simulink model "hyd_hyb_2_opt_cont_test_new_1_col_ab"  (Simulink 7.7)
settings: Solver=ode14x  StartTime=0  StopTime=750  FixedStep=0.01  MaxStep=.4  RelTol=1e-8
InitFcn:
  | const_3
values from neighbouring files (symbols this model uses):
  Af = 6.3    [brk_enrg.m]    (also in brk_enrg_1.m)
  BSFC_906_205 = [3.5684 4.3466 5.1668 6.0769 6.988 7.8992 8.8104 9.7336 10.8548 11.9809 13.1112 14.2571 ... (663 elements, 39x17)]    [BSFC_map_906_205.mat]
  Cd = 0.55    [brk_enrg.m]    (also in brk_enrg_1.m)
  den = 1.202    [brk_enrg.m]    (also in brk_enrg_1.m)
  fr = 0.01    [brk_enrg.m]    (also in brk_enrg_1.m)
undefined symbols (not defined in the file or in its folder): clutchRise, down_spd_2, down_spd_3, down_spd_4, down_spd_c, fuel_density, fuz_wgt, gearshift, I_wheel, Idle_spd_eng_906, M1_hydr, p_v_15, pmax_125, qv_125, Rw, sec_eng_sfc_map, T_max_906, T_min, TC_spd_ratio_max, torque_convertor, Twait, up_spd_1, up_spd_2, up_spd_3, up_spd_c, v_p_10, v_p_15, v_p_5
libraries: neural x2963, fl_lib x43, nesl_utility x30, sdl_lib x14, simulink x4, simulink_need_slupdate x1
(part 72 of 72)

== system hyd_hyb_2_opt_cont_test_new_1_col_ab/controller1/optimized controller - pm/SOC upper 0.5/SOC upper 0.6/SOC upper 0.7/SOC upper 0.8/net_pm_col_ab_89_b/Layer 1/IW{1,1} ==
  "pd{1,1}" : Inport  PortDimensions=2
      mask Display: e = 0.06;c1 = [120 230 180]/255;c2 = ([120 230 180]+0)/(256+128);c3 = ([120 230 180]+128)/(256+128);patch([0 1 1 0],[0 0 1 1],c1);patch([0 1 1 1-e 1-e 0+e],[0 0 1 1-e 0+e 0+e],c2);patch([0 0 1 1-e 0+e 0+e],[0 1 1 1-e 1-e 0+e],c3);plot([0 1 1 0 0],[0 0 1 1 0]);
  "IW{1,1}(1,:)'" : Constant  Value=[-7.5247531718272755;-6.6194856288513249]
      mask Display: e = 0.06;c1 = [120 150 230]/255;c2 = ([120 150 230]+0)/383;c3 = ([120 150 230]+128)/383;patch([0 1 1 0],[0 0 1 1],c1);patch([0 1 1 1-e 1-e 0+e],[0 0 1 1-e 0+e 0+e],c2);patch([0 0 1 1-e 0+e 0+e],[0 1 1 1-e 1-e 0+e],c3);plot([0 1 1 0 0],[0 0 1 1 0]);disp('Weights');
  "IW{1,1}(10,:)'" : Constant  Value=[-0.6823961158945111;-10.925155075088925]
      mask Display: e = 0.06;c1 = [120 150 230]/255;c2 = ([120 150 230]+0)/383;c3 = ([120 150 230]+128)/383;patch([0 1 1 0],[0 0 1 1],c1);patch([0 1 1 1-e 1-e 0+e],[0 0 1 1-e 0+e 0+e],c2);patch([0 0 1 1-e 0+e 0+e],[0 1 1 1-e 1-e 0+e],c3);plot([0 1 1 0 0],[0 0 1 1 0]);disp('Weights');
  "IW{1,1}(11,:)'" : Constant  Value=[10.664799059669335;0.99738837220633847]
      mask Display: e = 0.06;c1 = [120 150 230]/255;c2 = ([120 150 230]+0)/383;c3 = ([120 150 230]+128)/383;patch([0 1 1 0],[0 0 1 1],c1);patch([0 1 1 1-e 1-e 0+e],[0 0 1 1-e 0+e 0+e],c2);patch([0 0 1 1-e 0+e 0+e],[0 1 1 1-e 1-e 0+e],c3);plot([0 1 1 0 0],[0 0 1 1 0]);disp('Weights');
  "IW{1,1}(12,:)'" : Constant  Value=[1.8521265658009105;12.123277674207074]
      mask Display: e = 0.06;c1 = [120 150 230]/255;c2 = ([120 150 230]+0)/383;c3 = ([120 150 230]+128)/383;patch([0 1 1 0],[0 0 1 1],c1);patch([0 1 1 1-e 1-e 0+e],[0 0 1 1-e 0+e 0+e],c2);patch([0 0 1 1-e 0+e 0+e],[0 1 1 1-e 1-e 0+e],c3);plot([0 1 1 0 0],[0 0 1 1 0]);disp('Weights');
  "IW{1,1}(13,:)'" : Constant  Value=[9.8761699255633211;8.8585443365510503]
      mask Display: e = 0.06;c1 = [120 150 230]/255;c2 = ([120 150 230]+0)/383;c3 = ([120 150 230]+128)/383;patch([0 1 1 0],[0 0 1 1],c1);patch([0 1 1 1-e 1-e 0+e],[0 0 1 1-e 0+e 0+e],c2);patch([0 0 1 1-e 0+e 0+e],[0 1 1 1-e 1-e 0+e],c3);plot([0 1 1 0 0],[0 0 1 1 0]);disp('Weights');
  "IW{1,1}(14,:)'" : Constant  Value=[-5.3819119390644117;-7.2068768579593456]
      mask Display: e = 0.06;c1 = [120 150 230]/255;c2 = ([120 150 230]+0)/383;c3 = ([120 150 230]+128)/383;patch([0 1 1 0],[0 0 1 1],c1);patch([0 1 1 1-e 1-e 0+e],[0 0 1 1-e 0+e 0+e],c2);patch([0 0 1 1-e 0+e 0+e],[0 1 1 1-e 1-e 0+e],c3);plot([0 1 1 0 0],[0 0 1 1 0]);disp('Weights');
  "IW{1,1}(2,:)'" : Constant  Value=[3.4569355142303508;-9.9292384009218217]
      mask Display: e = 0.06;c1 = [120 150 230]/255;c2 = ([120 150 230]+0)/383;c3 = ([120 150 230]+128)/383;patch([0 1 1 0],[0 0 1 1],c1);patch([0 1 1 1-e 1-e 0+e],[0 0 1 1-e 0+e 0+e],c2);patch([0 0 1 1-e 0+e 0+e],[0 1 1 1-e 1-e 0+e],c3);plot([0 1 1 0 0],[0 0 1 1 0]);disp('Weights');
  "IW{1,1}(3,:)'" : Constant  Value=[-12.547076601117634;-1.3578183381190847]
      mask Display: e = 0.06;c1 = [120 150 230]/255;c2 = ([120 150 230]+0)/383;c3 = ([120 150 230]+128)/383;patch([0 1 1 0],[0 0 1 1],c1);patch([0 1 1 1-e 1-e 0+e],[0 0 1 1-e 0+e 0+e],c2);patch([0 0 1 1-e 0+e 0+e],[0 1 1 1-e 1-e 0+e],c3);plot([0 1 1 0 0],[0 0 1 1 0]);disp('Weights');
  "IW{1,1}(4,:)'" : Constant  Value=[-8.5225171072598229;-5.570962150913628]
      mask Display: e = 0.06;c1 = [120 150 230]/255;c2 = ([120 150 230]+0)/383;c3 = ([120 150 230]+128)/383;patch([0 1 1 0],[0 0 1 1],c1);patch([0 1 1 1-e 1-e 0+e],[0 0 1 1-e 0+e 0+e],c2);patch([0 0 1 1-e 0+e 0+e],[0 1 1 1-e 1-e 0+e],c3);plot([0 1 1 0 0],[0 0 1 1 0]);disp('Weights');
  "IW{1,1}(5,:)'" : Constant  Value=[-10.165810742362757;-2.8900949275636489]
      mask Display: e = 0.06;c1 = [120 150 230]/255;c2 = ([120 150 230]+0)/383;c3 = ([120 150 230]+128)/383;patch([0 1 1 0],[0 0 1 1],c1);patch([0 1 1 1-e 1-e 0+e],[0 0 1 1-e 0+e 0+e],c2);patch([0 0 1 1-e 0+e 0+e],[0 1 1 1-e 1-e 0+e],c3);plot([0 1 1 0 0],[0 0 1 1 0]);disp('Weights');
  "IW{1,1}(6,:)'" : Constant  Value=[-2.1735501686188834;-10.275369821335833]
      mask Display: e = 0.06;c1 = [120 150 230]/255;c2 = ([120 150 230]+0)/383;c3 = ([120 150 230]+128)/383;patch([0 1 1 0],[0 0 1 1],c1);patch([0 1 1 1-e 1-e 0+e],[0 0 1 1-e 0+e 0+e],c2);patch([0 0 1 1-e 0+e 0+e],[0 1 1 1-e 1-e 0+e],c3);plot([0 1 1 0 0],[0 0 1 1 0]);disp('Weights');
  "IW{1,1}(7,:)'" : Constant  Value=[-9.29204133005614;-5.9530925476551584]
      mask Display: e = 0.06;c1 = [120 150 230]/255;c2 = ([120 150 230]+0)/383;c3 = ([120 150 230]+128)/383;patch([0 1 1 0],[0 0 1 1],c1);patch([0 1 1 1-e 1-e 0+e],[0 0 1 1-e 0+e 0+e],c2);patch([0 0 1 1-e 0+e 0+e],[0 1 1 1-e 1-e 0+e],c3);plot([0 1 1 0 0],[0 0 1 1 0]);disp('Weights');
  "IW{1,1}(8,:)'" : Constant  Value=[8.790230610730708;6.6353593624284661]
      mask Display: e = 0.06;c1 = [120 150 230]/255;c2 = ([120 150 230]+0)/383;c3 = ([120 150 230]+128)/383;patch([0 1 1 0],[0 0 1 1],c1);patch([0 1 1 1-e 1-e 0+e],[0 0 1 1-e 0+e 0+e],c2);patch([0 0 1 1-e 0+e 0+e],[0 1 1 1-e 1-e 0+e],c3);plot([0 1 1 0 0],[0 0 1 1 0]);disp('Weights');
  "IW{1,1}(9,:)'" : Constant  Value=[6.7949067036882491;-8.3979254167496382]
      mask Display: e = 0.06;c1 = [120 150 230]/255;c2 = ([120 150 230]+0)/383;c3 = ([120 150 230]+128)/383;patch([0 1 1 0],[0 0 1 1],c1);patch([0 1 1 1-e 1-e 0+e],[0 0 1 1-e 0+e 0+e],c2);patch([0 0 1 1-e 0+e 0+e],[0 1 1 1-e 1-e 0+e],c3);plot([0 1 1 0 0],[0 0 1 1 0]);disp('Weights');
  Mux : Mux  Inputs=14
      mask Display: e = 0.06;c1 = [120 150 230]/255;c2 = ([120 150 230]+0)/383;c3 = ([120 150 230]+128)/383;patch([0 1 1 0],[0 0 1 1],c1);patch([0 1 1 1-e 1-e 0+e],[0 0 1 1-e 0+e 0+e],c2);patch([0 0 1 1-e 0+e 0+e],[0 1 1 1-e 1-e 0+e],c3);plot([0 1 1 0 0],[0 0 1 1 0]);disp('Mux');
  dotprod{1..14} (14 alike) : neural/Weight Functions/dotprod  SystemSampleTime=-1
  "iz{1,1}" : Outport
      mask Display: e = 0.06;c1 = [120 230 180]/255;c2 = ([120 230 180]+0)/(256+128);c3 = ([120 230 180]+128)/(256+128);patch([0 1 1 0],[0 0 1 1],c1);patch([0 1 1 1-e 1-e 0+e],[0 0 1 1-e 0+e 0+e],c2);patch([0 0 1 1-e 0+e 0+e],[0 1 1 1-e 1-e 0+e],c3);plot([0 1 1 0 0],[0 0 1 1 0]);
  wires:
  "IW{1,1}(1,:)'"/1 -> dotprod1/1
  "pd{1,1}"/1 -> dotprod1/2, dotprod2/2, dotprod3/2, dotprod4/2, dotprod5/2, dotprod6/2, dotprod7/2, dotprod8/2, dotprod9/2, dotprod10/2, dotprod11/2, dotprod12/2, dotprod13/2, dotprod14/2
  dotprod1/1 -> Mux/1
  "IW{1,1}(2,:)'"/1 -> dotprod2/1
  dotprod2/1 -> Mux/2
  "IW{1,1}(3,:)'"/1 -> dotprod3/1
  dotprod3/1 -> Mux/3
  "IW{1,1}(4,:)'"/1 -> dotprod4/1
  dotprod4/1 -> Mux/4
  "IW{1,1}(5,:)'"/1 -> dotprod5/1
  dotprod5/1 -> Mux/5
  "IW{1,1}(6,:)'"/1 -> dotprod6/1
  dotprod6/1 -> Mux/6
  "IW{1,1}(7,:)'"/1 -> dotprod7/1
  dotprod7/1 -> Mux/7
  "IW{1,1}(8,:)'"/1 -> dotprod8/1
  dotprod8/1 -> Mux/8
  "IW{1,1}(9,:)'"/1 -> dotprod9/1
  dotprod9/1 -> Mux/9
  "IW{1,1}(10,:)'"/1 -> dotprod10/1
  dotprod10/1 -> Mux/10
  "IW{1,1}(11,:)'"/1 -> dotprod11/1
  dotprod11/1 -> Mux/11
  "IW{1,1}(12,:)'"/1 -> dotprod12/1
  dotprod12/1 -> Mux/12
  "IW{1,1}(13,:)'"/1 -> dotprod13/1
  dotprod13/1 -> Mux/13
  "IW{1,1}(14,:)'"/1 -> dotprod14/1
  dotprod14/1 -> Mux/14
  Mux/1 -> "iz{1,1}"/1

== system hyd_hyb_2_opt_cont_test_new_1_col_ab/controller1/optimized controller - pm/SOC upper 0.5/SOC upper 0.6/SOC upper 0.7/SOC upper 0.8/net_pm_col_ab_89_b/Layer 2/Delays 1 ==
  "p{2}" : Inport  PortDimensions=14
      mask Display: e = 0.06;c1 = [120 230 180]/255;c2 = ([120 230 180]+0)/(256+128);c3 = ([120 230 180]+128)/(256+128);patch([0 1 1 0],[0 0 1 1],c1);patch([0 1 1 1-e 1-e 0+e],[0 0 1 1-e 0+e 0+e],c2);patch([0 0 1 1-e 0+e 0+e],[0 1 1 1-e 1-e 0+e],c3);plot([0 1 1 0 0],[0 0 1 1 0]);
  mux : Mux  Inputs=1
      mask Display: e = 0.06;c1 = [120 150 230]/255;c2 = ([120 150 230]+0)/383;c3 = ([120 150 230]+128)/383;patch([0 1 1 0],[0 0 1 1],c1);patch([0 1 1 1-e 1-e 0+e],[0 0 1 1-e 0+e 0+e],c2);patch([0 0 1 1-e 0+e 0+e],[0 1 1 1-e 1-e 0+e],c3);plot([0 1 1 0 0],[0 0 1 1 0]);disp('Mux')
  "pd{2,1}" : Outport
      mask Display: e = 0.06;c1 = [120 230 180]/255;c2 = ([120 230 180]+0)/(256+128);c3 = ([120 230 180]+128)/(256+128);patch([0 1 1 0],[0 0 1 1],c1);patch([0 1 1 1-e 1-e 0+e],[0 0 1 1-e 0+e 0+e],c2);patch([0 0 1 1-e 0+e 0+e],[0 1 1 1-e 1-e 0+e],c3);plot([0 1 1 0 0],[0 0 1 1 0]);
  wires:
  "p{2}"/1 -> mux/1
  mux/1 -> "pd{2,1}"/1

== system hyd_hyb_2_opt_cont_test_new_1_col_ab/controller1/optimized controller - pm/SOC upper 0.5/SOC upper 0.6/SOC upper 0.7/SOC upper 0.8/net_pm_col_ab_89_b/Layer 2/LW{2,1} ==
  "ad{2,1}" : Inport  PortDimensions=14
      mask Display: e = 0.06;c1 = [120 230 180]/255;c2 = ([120 230 180]+0)/(256+128);c3 = ([120 230 180]+128)/(256+128);patch([0 1 1 0],[0 0 1 1],c1);patch([0 1 1 1-e 1-e 0+e],[0 0 1 1-e 0+e 0+e],c2);patch([0 0 1 1-e 0+e 0+e],[0 1 1 1-e 1-e 0+e],c3);plot([0 1 1 0 0],[0 0 1 1 0]);
  "IW{2,1}(1,:)'" : Constant  Value=[-2.4172362230861912;0.11897161735180822;1.0748128173288254;1.8399688966148295;-1.4152398719051704;1.2831233160485713;0.66619502504356864;-3.7040294434188823;-1.6806920562993684;1.4872763390073449;0.22853571508898307;2.8622448895932617;1.6734974110640288;2.3644811190421393]
      mask Display: e = 0.06;c1 = [120 150 230]/255;c2 = ([120 150 230]+0)/383;c3 = ([120 150 230]+128)/383;patch([0 1 1 0],[0 0 1 1],c1);patch([0 1 1 1-e 1-e 0+e],[0 0 1 1-e 0+e 0+e],c2);patch([0 0 1 1-e 0+e 0+e],[0 1 1 1-e 1-e 0+e],c3);plot([0 1 1 0 0],[0 0 1 1 0]);disp('weights');
  "IW{2,1}(10,:)'" : Constant  Value=[2.1725003018942353;-2.9655421023585693;-1.9087526907654899;0.066652972634019494;-1.1505504360832728;-2.348205137749996;-2.6986676089208288;-3.134462355153997;-2.4105504942557419;-3.5078854741284391;-0.73741384502139795;-0.65533890123243521;-1.9470593645380398;-2.4696809181554595]
      mask Display: e = 0.06;c1 = [120 150 230]/255;c2 = ([120 150 230]+0)/383;c3 = ([120 150 230]+128)/383;patch([0 1 1 0],[0 0 1 1],c1);patch([0 1 1 1-e 1-e 0+e],[0 0 1 1-e 0+e 0+e],c2);patch([0 0 1 1-e 0+e 0+e],[0 1 1 1-e 1-e 0+e],c3);plot([0 1 1 0 0],[0 0 1 1 0]);disp('weights');
  "IW{2,1}(11,:)'" : Constant  Value=[-2.5933643387457002;2.5545627202222185;2.211227347756926;2.4888386205217095;-2.2126597028366959;-1.6982454335324346;1.1168846576098335;-1.6225169470611134;0.065669659624881027;-1.6044919910126278;-2.1834160484889371;-1.4830708033907178;-2.6046033852372132;0.60418726384841592]
      mask Display: e = 0.06;c1 = [120 150 230]/255;c2 = ([120 150 230]+0)/383;c3 = ([120 150 230]+128)/383;patch([0 1 1 0],[0 0 1 1],c1);patch([0 1 1 1-e 1-e 0+e],[0 0 1 1-e 0+e 0+e],c2);patch([0 0 1 1-e 0+e 0+e],[0 1 1 1-e 1-e 0+e],c3);plot([0 1 1 0 0],[0 0 1 1 0]);disp('weights');
  "IW{2,1}(12,:)'" : Constant  Value=[0.41776138082884423;1.1616575613709048;3.1624551472126785;0.15153831972678841;3.3792766234437628;-0.54551506204933842;2.4872961168069634;-3.9052903976701483;0.74793484381282727;-1.6510591359866753;-2.0990644470699142;0.54976895404716219;-0.37910567236226694;-0.6303083536203159]
      mask Display: e = 0.06;c1 = [120 150 230]/255;c2 = ([120 150 230]+0)/383;c3 = ([120 150 230]+128)/383;patch([0 1 1 0],[0 0 1 1],c1);patch([0 1 1 1-e 1-e 0+e],[0 0 1 1-e 0+e 0+e],c2);patch([0 0 1 1-e 0+e 0+e],[0 1 1 1-e 1-e 0+e],c3);plot([0 1 1 0 0],[0 0 1 1 0]);disp('weights');
  "IW{2,1}(13,:)'" : Constant  Value=[0.50709546875704448;-0.58821055155072577;1.398613739072422;-0.72546151926088176;3.5564437154025641;-2.0994820022338758;-2.283904232447445;-2.4380752490589734;-1.6905474514643934;-0.53213646513220425;1.7943020441130921;0.89862208959707834;-0.070993543097669073;-3.0005230302219879]
      mask Display: e = 0.06;c1 = [120 150 230]/255;c2 = ([120 150 230]+0)/383;c3 = ([120 150 230]+128)/383;patch([0 1 1 0],[0 0 1 1],c1);patch([0 1 1 1-e 1-e 0+e],[0 0 1 1-e 0+e 0+e],c2);patch([0 0 1 1-e 0+e 0+e],[0 1 1 1-e 1-e 0+e],c3);plot([0 1 1 0 0],[0 0 1 1 0]);disp('weights');
  "IW{2,1}(14,:)'" : Constant  Value=[3.534457099161485;-4.1320542418396951;0.078739257540870883;1.5905610535918537;-0.432799364806007;-1.7908582971400278;2.5462888504794305;-2.1943763662402609;-1.5679308424706604;1.5474481909029081;-3.0678549358659484;1.5855662866325959;0.50132812925635606;0.16411991606596901]
      mask Display: e = 0.06;c1 = [120 150 230]/255;c2 = ([120 150 230]+0)/383;c3 = ([120 150 230]+128)/383;patch([0 1 1 0],[0 0 1 1],c1);patch([0 1 1 1-e 1-e 0+e],[0 0 1 1-e 0+e 0+e],c2);patch([0 0 1 1-e 0+e 0+e],[0 1 1 1-e 1-e 0+e],c3);plot([0 1 1 0 0],[0 0 1 1 0]);disp('weights');
  "IW{2,1}(15,:)'" : Constant  Value=[2.1918405757272117;-0.47254642248355;3.2715136126520816;-2.9310413818595911;-1.9953384334849096;-1.4827954973194841;-0.99979929531938083;0.78806692216980767;-1.0384814338569501;1.3010047138362109;-3.602428288330187;-2.6535963496017652;1.0906482577921521;-2.1302692121272546]
      mask Display: e = 0.06;c1 = [120 150 230]/255;c2 = ([120 150 230]+0)/383;c3 = ([120 150 230]+128)/383;patch([0 1 1 0],[0 0 1 1],c1);patch([0 1 1 1-e 1-e 0+e],[0 0 1 1-e 0+e 0+e],c2);patch([0 0 1 1-e 0+e 0+e],[0 1 1 1-e 1-e 0+e],c3);plot([0 1 1 0 0],[0 0 1 1 0]);disp('weights');
  "IW{2,1}(16,:)'" : Constant  Value=[2.3129326464840299;-2.0759890119479349;-2.159520002281988;-2.7820723558551594;-2.4589697299428566;0.31676292483825658;-2.3172182178389007;-2.7478027659474269;-0.83557242358878425;0.20241259683531546;2.6884454714948474;-1.3430817982353236;1.3997111038371952;0.45903833156184748]
      mask Display: e = 0.06;c1 = [120 150 230]/255;c2 = ([120 150 230]+0)/383;c3 = ([120 150 230]+128)/383;patch([0 1 1 0],[0 0 1 1],c1);patch([0 1 1 1-e 1-e 0+e],[0 0 1 1-e 0+e 0+e],c2);patch([0 0 1 1-e 0+e 0+e],[0 1 1 1-e 1-e 0+e],c3);plot([0 1 1 0 0],[0 0 1 1 0]);disp('weights');
  "IW{2,1}(2,:)'" : Constant  Value=[3.3571627506024888;2.0531103212770732;-4.7326889763830531;1.4535873534232926;-1.3728165237721428;0.67156133990091338;-0.18650732883396581;0.157346870005002;-1.5887365286589701;-2.6485348216407436;-0.76688793985326076;0.58408693846552506;0.48952881272880633;-2.5090041310305251]
      mask Display: e = 0.06;c1 = [120 150 230]/255;c2 = ([120 150 230]+0)/383;c3 = ([120 150 230]+128)/383;patch([0 1 1 0],[0 0 1 1],c1);patch([0 1 1 1-e 1-e 0+e],[0 0 1 1-e 0+e 0+e],c2);patch([0 0 1 1-e 0+e 0+e],[0 1 1 1-e 1-e 0+e],c3);plot([0 1 1 0 0],[0 0 1 1 0]);disp('weights');
  "IW{2,1}(3,:)'" : Constant  Value=[-3.3879347647665794;-1.9854890023165639;0.56622843122448996;-2.2879571624728885;-0.75509616648893074;2.0245695790829532;-1.3640317952033443;0.48972191111965918;-1.5585028032341999;1.4861315866386742;-3.5316332686162641;-1.1648123578319018;0.36365121331864425;3.0762324456456707]
      mask Display: e = 0.06;c1 = [120 150 230]/255;c2 = ([120 150 230]+0)/383;c3 = ([120 150 230]+128)/383;patch([0 1 1 0],[0 0 1 1],c1);patch([0 1 1 1-e 1-e 0+e],[0 0 1 1-e 0+e 0+e],c2);patch([0 0 1 1-e 0+e 0+e],[0 1 1 1-e 1-e 0+e],c3);plot([0 1 1 0 0],[0 0 1 1 0]);disp('weights');
  "IW{2,1}(4,:)'" : Constant  Value=[2.0211344960852595;0.014466862357254065;-2.3520926079962257;-3.6540842564017879;-1.7545345096539569;-1.0256918319784438;-1.4359839895800264;0.48210994853512351;1.8817986588659288;-3.3567643773224316;1.8165313385769932;-1.6581875347860515;2.3254757075531396;-0.20817622227885704]
      mask Display: e = 0.06;c1 = [120 150 230]/255;c2 = ([120 150 230]+0)/383;c3 = ([120 150 230]+128)/383;patch([0 1 1 0],[0 0 1 1],c1);patch([0 1 1 1-e 1-e 0+e],[0 0 1 1-e 0+e 0+e],c2);patch([0 0 1 1-e 0+e 0+e],[0 1 1 1-e 1-e 0+e],c3);plot([0 1 1 0 0],[0 0 1 1 0]);disp('weights');
  "IW{2,1}(5,:)'" : Constant  Value=[-1.0861135293511195;1.4553057473806696;2.8448215015609346;0.48870418928621384;0.40571620989534984;2.9214425056822142;2.3436993698278039;2.2800169237776311;-2.2459638340134034;-0.80669068663328081;1.3554178196295357;-2.3403494142485592;2.0382971734780395;-1.6272344659045463]
      mask Display: e = 0.06;c1 = [120 150 230]/255;c2 = ([120 150 230]+0)/383;c3 = ([120 150 230]+128)/383;patch([0 1 1 0],[0 0 1 1],c1);patch([0 1 1 1-e 1-e 0+e],[0 0 1 1-e 0+e 0+e],c2);patch([0 0 1 1-e 0+e 0+e],[0 1 1 1-e 1-e 0+e],c3);plot([0 1 1 0 0],[0 0 1 1 0]);disp('weights');
  "IW{2,1}(6,:)'" : Constant  Value=[-0.008082877805686858;-2.3669919588881019;-2.6441715959511973;1.8579481999498177;0.071169021684155287;1.4061716716917096;-1.1481218940025828;-0.97052348941325428;-3.577040385677853;0.51406906683034226;0.01950463106911551;-3.7921968706020568;-1.818558898387826;-2.7545403616326598]
      mask Display: e = 0.06;c1 = [120 150 230]/255;c2 = ([120 150 230]+0)/383;c3 = ([120 150 230]+128)/383;patch([0 1 1 0],[0 0 1 1],c1);patch([0 1 1 1-e 1-e 0+e],[0 0 1 1-e 0+e 0+e],c2);patch([0 0 1 1-e 0+e 0+e],[0 1 1 1-e 1-e 0+e],c3);plot([0 1 1 0 0],[0 0 1 1 0]);disp('weights');
  "IW{2,1}(7,:)'" : Constant  Value=[1.0333699164074051;-4.7564025578379425;-2.5133333071971178;-2.6180758758106304;0.085090001844874794;1.6470545889105794;1.4540450259717943;2.5401452806549703;1.4982535749539914;1.1962749672372244;-2.2655375434754998;-1.7715941023733006;5.718831378290016;1.8215496716964774]
      mask Display: e = 0.06;c1 = [120 150 230]/255;c2 = ([120 150 230]+0)/383;c3 = ([120 150 230]+128)/383;patch([0 1 1 0],[0 0 1 1],c1);patch([0 1 1 1-e 1-e 0+e],[0 0 1 1-e 0+e 0+e],c2);patch([0 0 1 1-e 0+e 0+e],[0 1 1 1-e 1-e 0+e],c3);plot([0 1 1 0 0],[0 0 1 1 0]);disp('weights');
  "IW{2,1}(8,:)'" : Constant  Value=[2.0791549180903077;1.4892201355287769;-0.39167571512722738;0.051653534972530939;-2.7649325329615704;2.0087726350981159;-1.7442913253058761;-1.4884105014468387;-2.2369433337009186;1.3307183661194537;1.5613520863184913;0.057796536682294647;2.7089543036083175;-2.7700457021943299]
      mask Display: e = 0.06;c1 = [120 150 230]/255;c2 = ([120 150 230]+0)/383;c3 = ([120 150 230]+128)/383;patch([0 1 1 0],[0 0 1 1],c1);patch([0 1 1 1-e 1-e 0+e],[0 0 1 1-e 0+e 0+e],c2);patch([0 0 1 1-e 0+e 0+e],[0 1 1 1-e 1-e 0+e],c3);plot([0 1 1 0 0],[0 0 1 1 0]);disp('weights');
  "IW{2,1}(9,:)'" : Constant  Value=[0.049188324138078152;-3.3115329395128148;-0.5578332459164661;1.3563010051159943;0.53362556636015124;-2.4614034003523892;-3.3448890818363246;-1.9706522895258005;-1.6399413145238655;1.038444206126417;2.3792391510764315;0.46577693918800517;2.3172844283256344;1.8966635629755288]
      mask Display: e = 0.06;c1 = [120 150 230]/255;c2 = ([120 150 230]+0)/383;c3 = ([120 150 230]+128)/383;patch([0 1 1 0],[0 0 1 1],c1);patch([0 1 1 1-e 1-e 0+e],[0 0 1 1-e 0+e 0+e],c2);patch([0 0 1 1-e 0+e 0+e],[0 1 1 1-e 1-e 0+e],c3);plot([0 1 1 0 0],[0 0 1 1 0]);disp('weights');
  Mux : Mux  Inputs=16
      mask Display: e = 0.06;c1 = [120 150 230]/255;c2 = ([120 150 230]+0)/383;c3 = ([120 150 230]+128)/383;patch([0 1 1 0],[0 0 1 1],c1);patch([0 1 1 1-e 1-e 0+e],[0 0 1 1-e 0+e 0+e],c2);patch([0 0 1 1-e 0+e 0+e],[0 1 1 1-e 1-e 0+e],c3);plot([0 1 1 0 0],[0 0 1 1 0]);disp('Mux');
  dotprod{1..16} (16 alike) : neural/Weight Functions/dotprod  SystemSampleTime=-1
  "lz{2,1}" : Outport
      mask Display: e = 0.06;c1 = [120 230 180]/255;c2 = ([120 230 180]+0)/(256+128);c3 = ([120 230 180]+128)/(256+128);patch([0 1 1 0],[0 0 1 1],c1);patch([0 1 1 1-e 1-e 0+e],[0 0 1 1-e 0+e 0+e],c2);patch([0 0 1 1-e 0+e 0+e],[0 1 1 1-e 1-e 0+e],c3);plot([0 1 1 0 0],[0 0 1 1 0]);
  wires:
  "IW{2,1}(1,:)'"/1 -> dotprod1/1
  "ad{2,1}"/1 -> dotprod1/2, dotprod2/2, dotprod3/2, dotprod4/2, dotprod5/2, dotprod6/2, dotprod7/2, dotprod8/2, dotprod9/2, dotprod10/2, dotprod11/2, dotprod12/2, dotprod13/2, dotprod14/2, dotprod15/2, dotprod16/2
  dotprod1/1 -> Mux/1
  "IW{2,1}(2,:)'"/1 -> dotprod2/1
  dotprod2/1 -> Mux/2
  "IW{2,1}(3,:)'"/1 -> dotprod3/1
  dotprod3/1 -> Mux/3
  "IW{2,1}(4,:)'"/1 -> dotprod4/1
  dotprod4/1 -> Mux/4
  "IW{2,1}(5,:)'"/1 -> dotprod5/1
  dotprod5/1 -> Mux/5
  "IW{2,1}(6,:)'"/1 -> dotprod6/1
  dotprod6/1 -> Mux/6
  "IW{2,1}(7,:)'"/1 -> dotprod7/1
  dotprod7/1 -> Mux/7
  "IW{2,1}(8,:)'"/1 -> dotprod8/1
  dotprod8/1 -> Mux/8
  "IW{2,1}(9,:)'"/1 -> dotprod9/1
  dotprod9/1 -> Mux/9
  "IW{2,1}(10,:)'"/1 -> dotprod10/1
  dotprod10/1 -> Mux/10
  "IW{2,1}(11,:)'"/1 -> dotprod11/1
  dotprod11/1 -> Mux/11
  "IW{2,1}(12,:)'"/1 -> dotprod12/1
  dotprod12/1 -> Mux/12
  "IW{2,1}(13,:)'"/1 -> dotprod13/1
  dotprod13/1 -> Mux/13
  "IW{2,1}(14,:)'"/1 -> dotprod14/1
  dotprod14/1 -> Mux/14
  "IW{2,1}(15,:)'"/1 -> dotprod15/1
  dotprod15/1 -> Mux/15
  "IW{2,1}(16,:)'"/1 -> dotprod16/1
  dotprod16/1 -> Mux/16
  Mux/1 -> "lz{2,1}"/1

== system hyd_hyb_2_opt_cont_test_new_1_col_ab/controller1/optimized controller - pm/SOC upper 0.5/SOC upper 0.6/SOC upper 0.7/SOC upper 0.8/net_pm_col_ab_89_b/Layer 3/Delays 1 ==
  "p{3}" : Inport  PortDimensions=16
      mask Display: e = 0.06;c1 = [120 230 180]/255;c2 = ([120 230 180]+0)/(256+128);c3 = ([120 230 180]+128)/(256+128);patch([0 1 1 0],[0 0 1 1],c1);patch([0 1 1 1-e 1-e 0+e],[0 0 1 1-e 0+e 0+e],c2);patch([0 0 1 1-e 0+e 0+e],[0 1 1 1-e 1-e 0+e],c3);plot([0 1 1 0 0],[0 0 1 1 0]);
  mux : Mux  Inputs=1
      mask Display: e = 0.06;c1 = [120 150 230]/255;c2 = ([120 150 230]+0)/383;c3 = ([120 150 230]+128)/383;patch([0 1 1 0],[0 0 1 1],c1);patch([0 1 1 1-e 1-e 0+e],[0 0 1 1-e 0+e 0+e],c2);patch([0 0 1 1-e 0+e 0+e],[0 1 1 1-e 1-e 0+e],c3);plot([0 1 1 0 0],[0 0 1 1 0]);disp('Mux')
  "pd{3,2}" : Outport
      mask Display: e = 0.06;c1 = [120 230 180]/255;c2 = ([120 230 180]+0)/(256+128);c3 = ([120 230 180]+128)/(256+128);patch([0 1 1 0],[0 0 1 1],c1);patch([0 1 1 1-e 1-e 0+e],[0 0 1 1-e 0+e 0+e],c2);patch([0 0 1 1-e 0+e 0+e],[0 1 1 1-e 1-e 0+e],c3);plot([0 1 1 0 0],[0 0 1 1 0]);
  wires:
  "p{3}"/1 -> mux/1
  mux/1 -> "pd{3,2}"/1

== system hyd_hyb_2_opt_cont_test_new_1_col_ab/controller1/optimized controller - pm/SOC upper 0.5/SOC upper 0.6/SOC upper 0.7/SOC upper 0.8/net_pm_col_ab_89_b/Layer 3/LW{3,2} ==
  "ad{3,2}" : Inport  PortDimensions=16
      mask Display: e = 0.06;c1 = [120 230 180]/255;c2 = ([120 230 180]+0)/(256+128);c3 = ([120 230 180]+128)/(256+128);patch([0 1 1 0],[0 0 1 1],c1);patch([0 1 1 1-e 1-e 0+e],[0 0 1 1-e 0+e 0+e],c2);patch([0 0 1 1-e 0+e 0+e],[0 1 1 1-e 1-e 0+e],c3);plot([0 1 1 0 0],[0 0 1 1 0]);
  "IW{3,2}(1,:)'" : Constant  Value=[1.8308209749369431;-2.8468718849331269;-1.067030689174822;-0.042338974513845169;1.6420445875199055;-1.1399489254026312;-3.7522789314646441;1.4282263650858786;2.2591512092929866;-1.9354481817470226;2.2204525511649029;-2.7376045889141962;0.58983272477592419;-3.0702187815380984;3.0377926079475768;-1.1748374068836354]
      mask Display: e = 0.06;c1 = [120 150 230]/255;c2 = ([120 150 230]+0)/383;c3 = ([120 150 230]+128)/383;patch([0 1 1 0],[0 0 1 1],c1);patch([0 1 1 1-e 1-e 0+e],[0 0 1 1-e 0+e 0+e],c2);patch([0 0 1 1-e 0+e 0+e],[0 1 1 1-e 1-e 0+e],c3);plot([0 1 1 0 0],[0 0 1 1 0]);disp('weights');
  Mux : Mux  Inputs=1
      mask Display: e = 0.06;c1 = [120 150 230]/255;c2 = ([120 150 230]+0)/383;c3 = ([120 150 230]+128)/383;patch([0 1 1 0],[0 0 1 1],c1);patch([0 1 1 1-e 1-e 0+e],[0 0 1 1-e 0+e 0+e],c2);patch([0 0 1 1-e 0+e 0+e],[0 1 1 1-e 1-e 0+e],c3);plot([0 1 1 0 0],[0 0 1 1 0]);disp('Mux');
  dotprod1 : neural/Weight Functions/dotprod  SystemSampleTime=-1
  "lz{3,2}" : Outport
      mask Display: e = 0.06;c1 = [120 230 180]/255;c2 = ([120 230 180]+0)/(256+128);c3 = ([120 230 180]+128)/(256+128);patch([0 1 1 0],[0 0 1 1],c1);patch([0 1 1 1-e 1-e 0+e],[0 0 1 1-e 0+e 0+e],c2);patch([0 0 1 1-e 0+e 0+e],[0 1 1 1-e 1-e 0+e],c3);plot([0 1 1 0 0],[0 0 1 1 0]);
  wires:
  "IW{3,2}(1,:)'"/1 -> dotprod1/1
  "ad{3,2}"/1 -> dotprod1/2
  dotprod1/1 -> Mux/1
  Mux/1 -> "lz{3,2}"/1

== stateflow chart driver/Chart ==
  state transmission state control [group]
    state Drive
      during: s=1;
    state Neutral
      during: s=0;
    (default) -> Neutral
    Neutral -> Drive : [cyc> 0]
    Drive -> Neutral : after(1,sec)[cyc== 0]
  data: cyc input double; speed input double; s output double

== stateflow chart Transmission1/transmission control/Chart1 ==
  state fourth
    entry: gear = 4;
  state third
    entry: gear = 3;
  state second
    entry: gear = 2;
  state first
    entry: TC=1;
  state firstc
    entry: gear=1;
    entry: TC=0;
  second -> third [order 1] : after(Twait,tick)
      | [speed >= up_spd_2]
  first -> second [order 1] : after(Twait,tick)
      | [speed >= up_spd_1]
  third -> fourth [order 1] : after(Twait,tick)
      | [speed >= up_spd_3]
  firstc -> first : after(Twait,tick)
      | [speed >=up_spd_c]
  (default) -> firstc
  third -> second [order 2] : after(Twait,tick)
      | [speed < down_spd_3]
  fourth -> third : after(Twait,tick)
      | [speed < down_spd_4]
  first -> firstc [order 2] : after(Twait,tick)
      | [speed < down_spd_c]
  second -> first [order 2] : after(Twait,tick)
      | [speed < down_spd_2]
  data: speed input double; gear output double; TC output double; down_spd_4 parameter double; down_spd_2 parameter double; down_spd_3 parameter double; up_spd_1 parameter double; up_spd_2 parameter double; up_spd_3 parameter double; down_spd_c parameter double; up_spd_c parameter double; Twait parameter double

== matlab function controller1/MATLAB Function ==
  data: T_pm_1 input double; T_pm output double; T_eng output double; T_brk input double; T_dem input double; T_mech_brk output double; delta_p input double
function [T_pm,T_eng,T_mech_brk] = fcn(T_pm_1,T_brk,T_dem,delta_p)
if abs(T_dem)>0
    if abs(T_brk)>0.1
        T_eng=0;T_pm=T_pm_1;
        T_mech_brk=min(0,T_brk-T_pm_1);
    else    
        T_mech_brk=0;
        T_pm=min(T_pm_1,(delta_p*650/325));
        if delta_p<=140
            T_pm=0;
        end
        T_eng=max(0,T_dem-T_pm);
        if T_eng<100
            T_eng=0;
            T_pm=T_dem;
        end
    end
else
    T_pm=0;T_eng=0;T_mech_brk=0;
end
end

== stateflow chart hydraulic transmission/PM_hydraulic_machine /qv - pmax evaluation/Chart ==
  state state1
    during: u1=1;
  state state2
    during: u1=0;
  (default) -> state1
  state1 -> state2 : [soc <= soc_min]
  state2 -> state1 : [soc > 50]
  data: soc input double; soc_min input double; u1 output double

== matlab function controller1/optimized controller - pm/SOC under 0.5/Subsystem/MATLAB Function ==
  data: t input double; y output double; fuz_wgt input double
function y = fcn(t,fuz_wgt)
if t<=100
    y=fuz_wgt(4,1);
elseif t<=200
    y=fuz_wgt(4,2);
elseif t<=300
    y=fuz_wgt(4,3);
elseif t<=400
    y=fuz_wgt(4,4);
elseif t<=500
    y=fuz_wgt(4,5);
else
    y=fuz_wgt(4,6);
end
end

== matlab function controller1/optimized controller - pm/SOC under 0.5/Subsystem1/MATLAB Function ==
  data: t input double; y output double; fuz_wgt input double
function y = fcn(t,fuz_wgt)
if t<=100
    y=fuz_wgt(1,1);
elseif t<=200
    y=fuz_wgt(1,2);
elseif t<=300
    y=fuz_wgt(1,3);
elseif t<=400
    y=fuz_wgt(1,4);
elseif t<=500
    y=fuz_wgt(1,5);
else
    y=fuz_wgt(1,6);
end

== matlab function controller1/optimized controller - pm/SOC under 0.5/Subsystem2/MATLAB Function ==
  data: t input double; y output double; fuz_wgt input double
function y = fcn(t,fuz_wgt)
if t<=100
    y=fuz_wgt(2,1);
elseif t<=200
    y=fuz_wgt(2,2);
elseif t<=300
    y=fuz_wgt(2,3);
elseif t<=400
    y=fuz_wgt(2,4);
elseif t<=500
    y=fuz_wgt(2,5);
else
    y=fuz_wgt(2,6);
end

== matlab function controller1/optimized controller - pm/SOC under 0.5/Subsystem3/MATLAB Function ==
  data: t input double; y output double; fuz_wgt input double
function y = fcn(t,fuz_wgt)
if t<=100
    y=fuz_wgt(3,1);
elseif t<=200
    y=fuz_wgt(3,2);
elseif t<=300
    y=fuz_wgt(3,3);
elseif t<=400
    y=fuz_wgt(3,4);
elseif t<=500
    y=fuz_wgt(3,5);
else
    y=fuz_wgt(3,6);
end

== matlab function controller1/optimized controller - pm/SOC under 0.5/col_c_d1/MATLAB Function ==
  data: soc input double; u_0_4 output double; u_4_5 output double
function [u_0_4,u_4_5] = fcn(soc)
if soc<0.37
    u_0_4=1;
    u_4_5=0;
elseif soc<0.42
    u_0_4=(-1/0.05)*(soc-.42);
    u_4_5=(1/0.05)*(soc-.37);
else
    u_0_4=0;
    u_4_5=1;
end
end

== matlab function controller1/optimized controller - pm/SOC upper 0.5/SOC under 0.6/fuz_wgt_col_a_b/MATLAB Function ==
  data: t input double; y output double; fuz_wgt input double
function y = fcn(t,fuz_wgt)
if t<=100
    y=fuz_wgt(1,1);
elseif t<=200
    y=fuz_wgt(1,2);
elseif t<=300
    y=fuz_wgt(1,3);
elseif t<=400
    y=fuz_wgt(1,4);
elseif t<=500
    y=fuz_wgt(1,5);
else
    y=fuz_wgt(1,6);
end

== matlab function controller1/optimized controller - pm/SOC upper 0.5/SOC under 0.6/fuz_wgt_col_c_d/MATLAB Function ==
  data: t input double; y output double; fuz_wgt input double
function y = fcn(t,fuz_wgt)
if t<=100
    y=fuz_wgt(2,1);
elseif t<=200
    y=fuz_wgt(2,2);
elseif t<=300
    y=fuz_wgt(2,3);
elseif t<=400
    y=fuz_wgt(2,4);
elseif t<=500
    y=fuz_wgt(2,5);
else
    y=fuz_wgt(2,6);
end

== matlab function controller1/optimized controller - pm/SOC upper 0.5/SOC under 0.6/fuz_wgt_col_e_f/MATLAB Function ==
  data: t input double; y output double; fuz_wgt input double
function y = fcn(t,fuz_wgt)
if t<=100
    y=fuz_wgt(3,1);
elseif t<=200
    y=fuz_wgt(3,2);
elseif t<=300
    y=fuz_wgt(3,3);
elseif t<=400
    y=fuz_wgt(3,4);
elseif t<=500
    y=fuz_wgt(3,5);
else
    y=fuz_wgt(3,6);
end

== matlab function controller1/optimized controller - pm/SOC upper 0.5/SOC under 0.6/fuz_wgt_loc_rod/MATLAB Function ==
  data: t input double; y output double; fuz_wgt input double
function y = fcn(t,fuz_wgt)
if t<=100
    y=fuz_wgt(4,1);
elseif t<=200
    y=fuz_wgt(4,2);
elseif t<=300
    y=fuz_wgt(4,3);
elseif t<=400
    y=fuz_wgt(4,4);
elseif t<=500
    y=fuz_wgt(4,5);
else
    y=fuz_wgt(4,6);
end

== matlab function controller1/optimized controller - pm/SOC upper 0.5/SOC upper 0.6/SOC under 0.7/Subsystem/MATLAB Function ==
  data: t input double; y output double; fuz_wgt input double
function y = fcn(t,fuz_wgt)
if t<=100
    y=fuz_wgt(4,1);
elseif t<=200
    y=fuz_wgt(4,2);
elseif t<=300
    y=fuz_wgt(4,3);
elseif t<=400
    y=fuz_wgt(4,4);
elseif t<=500
    y=fuz_wgt(4,5);
else
    y=fuz_wgt(4,6);
end

== matlab function controller1/optimized controller - pm/SOC upper 0.5/SOC upper 0.6/SOC under 0.7/Subsystem1/MATLAB Function ==
  data: t input double; y output double; fuz_wgt input double
function y = fcn(t,fuz_wgt)
if t<=100
    y=fuz_wgt(1,1);
elseif t<=200
    y=fuz_wgt(1,2);
elseif t<=300
    y=fuz_wgt(1,3);
elseif t<=400
    y=fuz_wgt(1,4);
elseif t<=500
    y=fuz_wgt(1,5);
else
    y=fuz_wgt(1,6);
end

== matlab function controller1/optimized controller - pm/SOC upper 0.5/SOC upper 0.6/SOC under 0.7/Subsystem2/MATLAB Function ==
  data: t input double; y output double; fuz_wgt input double
function y = fcn(t,fuz_wgt)
if t<=100
    y=fuz_wgt(2,1);
elseif t<=200
    y=fuz_wgt(2,2);
elseif t<=300
    y=fuz_wgt(2,3);
elseif t<=400
    y=fuz_wgt(2,4);
elseif t<=500
    y=fuz_wgt(2,5);
else
    y=fuz_wgt(2,6);
end

== matlab function controller1/optimized controller - pm/SOC upper 0.5/SOC upper 0.6/SOC under 0.7/Subsystem3/MATLAB Function ==
  data: t input double; y output double; fuz_wgt input double
function y = fcn(t,fuz_wgt)
if t<=100
    y=fuz_wgt(3,1);
elseif t<=200
    y=fuz_wgt(3,2);
elseif t<=300
    y=fuz_wgt(3,3);
elseif t<=400
    y=fuz_wgt(3,4);
elseif t<=500
    y=fuz_wgt(3,5);
else
    y=fuz_wgt(3,6);
end

== matlab function controller1/optimized controller - pm/SOC upper 0.5/SOC upper 0.6/SOC upper 0.7/SOC under 0.8/Subsystem/MATLAB Function ==
  data: t input double; y output double; fuz_wgt input double
function y = fcn(t,fuz_wgt)
if t<=100
    y=fuz_wgt(4,1);
elseif t<=200
    y=fuz_wgt(4,2);
elseif t<=300
    y=fuz_wgt(4,3);
elseif t<=400
    y=fuz_wgt(4,4);
elseif t<=500
    y=fuz_wgt(4,5);
else
    y=fuz_wgt(4,6);
end

== matlab function controller1/optimized controller - pm/SOC upper 0.5/SOC upper 0.6/SOC upper 0.7/SOC under 0.8/Subsystem1/MATLAB Function ==
  data: t input double; y output double; fuz_wgt input double
function y = fcn(t,fuz_wgt)
if t<=100
    y=fuz_wgt(1,1);
elseif t<=200
    y=fuz_wgt(1,2);
elseif t<=300
    y=fuz_wgt(1,3);
elseif t<=400
    y=fuz_wgt(1,4);
elseif t<=500
    y=fuz_wgt(1,5);
else
    y=fuz_wgt(1,6);
end

== matlab function controller1/optimized controller - pm/SOC upper 0.5/SOC upper 0.6/SOC upper 0.7/SOC under 0.8/Subsystem2/MATLAB Function ==
  data: t input double; y output double; fuz_wgt input double
function y = fcn(t,fuz_wgt)
if t<=100
    y=fuz_wgt(2,1);
elseif t<=200
    y=fuz_wgt(2,2);
elseif t<=300
    y=fuz_wgt(2,3);
elseif t<=400
    y=fuz_wgt(2,4);
elseif t<=500
    y=fuz_wgt(2,5);
else
    y=fuz_wgt(2,6);
end

== matlab function controller1/optimized controller - pm/SOC upper 0.5/SOC upper 0.6/SOC upper 0.7/SOC under 0.8/Subsystem3/MATLAB Function ==
  data: t input double; y output double; fuz_wgt input double
function y = fcn(t,fuz_wgt)
if t<=100
    y=fuz_wgt(3,1);
elseif t<=200
    y=fuz_wgt(3,2);
elseif t<=300
    y=fuz_wgt(3,3);
elseif t<=400
    y=fuz_wgt(3,4);
elseif t<=500
    y=fuz_wgt(3,5);
else
    y=fuz_wgt(3,6);
end

== matlab function controller1/optimized controller - pm/SOC upper 0.5/SOC upper 0.6/SOC upper 0.7/SOC upper 0.8/Subsystem/MATLAB Function ==
  data: t input double; y output double; fuz_wgt input double
function y = fcn(t,fuz_wgt)
if t<=100
    y=fuz_wgt(4,1);
elseif t<=200
    y=fuz_wgt(4,2);
elseif t<=300
    y=fuz_wgt(4,3);
elseif t<=400
    y=fuz_wgt(4,4);
elseif t<=500
    y=fuz_wgt(4,5);
else
    y=fuz_wgt(4,6);
end

== matlab function controller1/optimized controller - pm/SOC upper 0.5/SOC upper 0.6/SOC upper 0.7/SOC upper 0.8/Subsystem1/MATLAB Function ==
  data: t input double; y output double; fuz_wgt input double
function y = fcn(t,fuz_wgt)
if t<=100
    y=fuz_wgt(1,1);
elseif t<=200
    y=fuz_wgt(1,2);
elseif t<=300
    y=fuz_wgt(1,3);
elseif t<=400
    y=fuz_wgt(1,4);
elseif t<=500
    y=fuz_wgt(1,5);
else
    y=fuz_wgt(1,6);
end

== matlab function controller1/optimized controller - pm/SOC upper 0.5/SOC upper 0.6/SOC upper 0.7/SOC upper 0.8/Subsystem2/MATLAB Function ==
  data: t input double; y output double; fuz_wgt input double
function y = fcn(t,fuz_wgt)
if t<=100
    y=fuz_wgt(2,1);
elseif t<=200
    y=fuz_wgt(2,2);
elseif t<=300
    y=fuz_wgt(2,3);
elseif t<=400
    y=fuz_wgt(2,4);
elseif t<=500
    y=fuz_wgt(2,5);
else
    y=fuz_wgt(2,6);
end

== matlab function controller1/optimized controller - pm/SOC upper 0.5/SOC upper 0.6/SOC upper 0.7/SOC upper 0.8/Subsystem3/MATLAB Function ==
  data: t input double; y output double; fuz_wgt input double
function y = fcn(t,fuz_wgt)
if t<=100
    y=fuz_wgt(3,1);
elseif t<=200
    y=fuz_wgt(3,2);
elseif t<=300
    y=fuz_wgt(3,3);
elseif t<=400
    y=fuz_wgt(3,4);
elseif t<=500
    y=fuz_wgt(3,5);
else
    y=fuz_wgt(3,6);
end
